simulink model "PrkgClimaActvMgr"  (R2022b) (continued)
== system PrkgClimaActvMgr == (continued)
  MemSaveFlgForBlwAftRun_MemSaveFlgForBlwAftRun : Outport  Port=18  OutDataTypeStr=Boolean  PortDimensions=1
  MemSaveFlgForHighTempCln_MemSaveFlgForHighTempCln : Outport  Port=19  OutDataTypeStr=Boolean  PortDimensions=1
  MemSaveFlgForHMISetEngReq_MemSaveFlgForHMISetEngReq : Outport  Port=20  OutDataTypeStr=Boolean  PortDimensions=1
  MemSaveFlgForParkVent_MemSaveFlgForParkVent : Outport  Port=21  OutDataTypeStr=Boolean  PortDimensions=1
  MemSaveFlgForParkVentActvCnt_MemSaveFlgForParkVentActvCnt : Outport  Port=22  OutDataTypeStr=Boolean  PortDimensions=1
  MemWrForBlwAftRun_MemWrForBlwAftRun : Outport  Port=23  OutDataTypeStr=Bus: MemRecForBlwAftRun  PortDimensions=1  BusOutputAsStruct=on
  MemWrForHighTempCln_MemWrForHighTempCln : Outport  Port=24  OutDataTypeStr=Bus: MemRecForHighTempCln  PortDimensions=1  BusOutputAsStruct=on
  MemWrForHMISetEngReq_MemWrForHMISetEngReq : Outport  Port=25  OutDataTypeStr=Bus: MemRecForHMISetEngReq  PortDimensions=1  BusOutputAsStruct=on
  MemWrForParkVent_MemWrForParkVent : Outport  Port=26  OutDataTypeStr=Bus: MemRecForParkVent  PortDimensions=1  BusOutputAsStruct=on
  MemWrForParkVentActvCnt_MemWrForParkVentActvCnt : Outport  Port=27  OutDataTypeStr=Bus: MemRecForParkVentCnt  PortDimensions=1  BusOutputAsStruct=on
  OGMAirSrcFnshd_OGMAirSrcFnshd : Outport  Port=28  OutDataTypeStr=Boolean  PortDimensions=1
  OGMAirSrcReq_OGMAirSrcReq : Outport  Port=29  OutDataTypeStr=Enum: OnOffNoReq  PortDimensions=1
  OGMAvlSts_OGMAvlSts : Outport  Port=30  OutDataTypeStr=Enum: OxygenSplyAvlSts  PortDimensions=1
  OGMReq_OGMReq : Outport  Port=31  OutDataTypeStr=Enum: OxygenSplyReq  PortDimensions=1
  ParkVentActv_ParkVentActv : Outport  Port=32  OutDataTypeStr=Boolean  PortDimensions=1
  ParkVentWkTi_ParkVentWkTi : Outport  Port=33  OutDataTypeStr=UInt8  PortDimensions=1
  PrkgClimaACReq_PrkgClimaACReq : Outport  Port=34  OutDataTypeStr=Enum: HmiCmptmtCoolgReq  PortDimensions=1
  PrkgClimaActvMgrVFCLDACClimaCtrl_PrkgClimaActvMgrVFCLDACClimaCtrl : Outport  Port=35  OutDataTypeStr=Boolean  PortDimensions=1
  PrkgClimaAirflowReqRe_PrkgClimaAirflowReqRe : Outport  Port=36  OutDataTypeStr=Enum: HmiHvacFanLvl  PortDimensions=1
  PrkgClimaEngStReqNgcc_PrkgClimaEngStReqNgcc : Outport  Port=37  OutDataTypeStr=Enum: OnOff1  PortDimensions=1
  PrkgClimaHVReq_PrkgClimaHVReq : Outport  Port=38  OutDataTypeStr=Enum: OnOff1  PortDimensions=1
  PrkgClimaOnReqRe_PrkgClimaOnReqRe : Outport  Port=39  OutDataTypeStr=Enum: OnOff1  PortDimensions=1
  PrkgCmftRlyReq_PrkgCmftRlyReq : Outport  Port=40  OutDataTypeStr=Boolean  PortDimensions=1
  PrkgOGMAirSrcActv_PrkgOGMAirSrcActv : Outport  Port=41  OutDataTypeStr=Boolean  PortDimensions=1
  PrkgVntFctn_PrkgVntFctn : Outport  Port=42  OutDataTypeStr=Boolean  PortDimensions=1
  PrvntWinFrz_PrvntWinFrz : Outport  Port=43  OutDataTypeStr=Boolean  PortDimensions=1
  PrvntWinFrzFnshd_PrvntWinFrzFnshd : Outport  Port=44  OutDataTypeStr=Boolean  PortDimensions=1
  RemActvSts_RemActvSts : Outport  Port=45  OutDataTypeStr=Bus: RemActvSts  PortDimensions=1  BusOutputAsStruct=on
  RemClimaFnshd1_RemClimaFnshd1 : Outport  Port=46  OutDataTypeStr=Boolean  PortDimensions=1
  RemClimaWarn_RemClimaWarn : Outport  Port=47  OutDataTypeStr=Enum: ClimaWarn  PortDimensions=1
  RemOxygenSplyActv_RemOxygenSplyActv : Outport  Port=48  OutDataTypeStr=Enum: OnOff1  PortDimensions=1
  RemOxygenSplyActvFaildNgcc_RemOxygenSplyActvFaildNgcc : Outport  Port=49  OutMin=0  OutMax=3  OutDataTypeStr=UInt2  PortDimensions=1
  RemOxygenSplyFnshd_RemOxygenSplyFnshd : Outport  Port=50  OutDataTypeStr=Boolean  PortDimensions=1
  RemOxygenSplyFnshd1_RemOxygenSplyFnshd1 : Outport  Port=51  OutDataTypeStr=Boolean  PortDimensions=1
  RemOxygenSplyWarn_RemOxygenSplyWarn : Outport  Port=52  OutDataTypeStr=Enum: ClimaWarn  PortDimensions=1
  RemSynchrnztnLcl_RemSynchrnztnLcl : Outport  Port=53  OutDataTypeStr=Bus: RemSynchrnztnLcl  PortDimensions=1  BusOutputAsStruct=on
  RemWinClsReq_RemWinClsReq : Outport  Port=54  OutDataTypeStr=Enum: NoReqOnOff  PortDimensions=1
  SeatClimaDurgClimaHmiSeatHeatgForRowThrdLe_SeatClimaDurgClimaHmiSeatHeatgForRowThrdLe : Outport  Port=55  OutDataTypeStr=Enum: SeatClimaLvl  PortDimensions=1
  SeatClimaDurgClimaHmiSeatHeatgForRowThrdRi_SeatClimaDurgClimaHmiSeatHeatgForRowThrdRi : Outport  Port=56  OutDataTypeStr=Enum: SeatClimaLvl  PortDimensions=1
  SeatClimaDurgClimaHmiSeatVentnForRowThrdLe_SeatClimaDurgClimaHmiSeatVentnForRowThrdLe : Outport  Port=57  OutDataTypeStr=Enum: SeatClimaLvl  PortDimensions=1
  SeatClimaDurgClimaHmiSeatVentnForRowThrdRi_SeatClimaDurgClimaHmiSeatVentnForRowThrdRi : Outport  Port=58  OutDataTypeStr=Enum: SeatClimaLvl  PortDimensions=1
  TelmDefrostFnshd_TelmDefrostFnshd : Outport  Port=59  OutDataTypeStr=Boolean  PortDimensions=1
  TelmSeatHeatDrvrFnshd_TelmSeatHeatDrvrFnshd : Outport  Port=60  OutDataTypeStr=Boolean  PortDimensions=1
  TelmSeatHeatPassFnshd_TelmSeatHeatPassFnshd : Outport  Port=61  OutDataTypeStr=Boolean  PortDimensions=1
  TelmSeatHeatSecLeFnshd_TelmSeatHeatSecLeFnshd : Outport  Port=62  OutDataTypeStr=Boolean  PortDimensions=1
  TelmSeatHeatSecRiFnshd_TelmSeatHeatSecRiFnshd : Outport  Port=63  OutDataTypeStr=Boolean  PortDimensions=1
  TelmSeatHeatThrdLeFnshd_TelmSeatHeatThrdLeFnshd : Outport  Port=64  OutDataTypeStr=Boolean  PortDimensions=1
  TelmSeatHeatThrdRiFnshd_TelmSeatHeatThrdRiFnshd : Outport  Port=65  OutDataTypeStr=Boolean  PortDimensions=1
  TelmSeatVentnDrvrFnshd_TelmSeatVentnDrvrFnshd : Outport  Port=66  OutDataTypeStr=Boolean  PortDimensions=1
  TelmSeatVentnPassFnshd_TelmSeatVentnPassFnshd : Outport  Port=67  OutDataTypeStr=Boolean  PortDimensions=1
  TelmSeatVentnSecLeFnshd_TelmSeatVentnSecLeFnshd : Outport  Port=68  OutDataTypeStr=Boolean  PortDimensions=1
  TelmSeatVentnSecRiFnshd_TelmSeatVentnSecRiFnshd : Outport  Port=69  OutDataTypeStr=Boolean  PortDimensions=1
  TelmSeatVentnThrdLeFnshd_TelmSeatVentnThrdLeFnshd : Outport  Port=70  OutDataTypeStr=Boolean  PortDimensions=1
  TelmSeatVentnThrdRiFnshd_TelmSeatVentnThrdRiFnshd : Outport  Port=71  OutDataTypeStr=Boolean  PortDimensions=1
  TelmSteerWhlHeatFnshd_TelmSteerWhlHeatFnshd : Outport  Port=72  OutDataTypeStr=Boolean  PortDimensions=1
  VMMChngNdMtnNgcc_VMMChngNdMtnNgcc : Outport  Port=73  OutDataTypeStr=Enum: OnOff1  PortDimensions=1
  VTMOverTProtection_VTMOverTProtection : Outport  Port=74  OutDataTypeStr=Boolean  PortDimensions=1
  VTMOverTProtectionFnshd_VTMOverTProtectionFnshd : Outport  Port=75  OutDataTypeStr=Boolean  PortDimensions=1
  BlwAftRunReq_BlwAftRunReq : Outport  Port=76  OutDataTypeStr=Boolean  PortDimensions=1
  BlwAftRunSts_BlwAftRunSts : Outport  Port=77  OutDataTypeStr=Boolean  PortDimensions=1
  BookTrvlClimaActv_BookTrvlClimaActv : Outport  Port=78  OutDataTypeStr=Enum: OnOff1  PortDimensions=1
  CdsParkgClimaActv_CdsParkgClimaActv : Outport  Port=79  OutDataTypeStr=Enum: OnOff1  PortDimensions=1
  ClimaActv_Ngcc_ClimaActv : Outport  Port=80  OutDataTypeStr=Enum: OnOff1  PortDimensions=1
  ClimaOvrHeatProActv_ClimaOvrHeatProActv : Outport  Port=81  OutDataTypeStr=Enum: OnOff1  PortDimensions=1
  ClimaOvrHeatProActvSts_ClimaOvrHeatProActvSts : Outport  Port=82  OutDataTypeStr=Enum: OnOff1  PortDimensions=1
  ClimaOvrHeatProWarn_ClimaOvrHeatProWarn : Outport  Port=83  OutDataTypeStr=Enum: ClimaOvrheatProWarnSts  PortDimensions=1
  ClimaOvrHeatPrtSts_ClimaOvrHeatPrtSts : Outport  Port=84  OutDataTypeStr=Enum: OnOff1  PortDimensions=1
  ClimaSts_ClimaSts : Outport  Port=85  OutDataTypeStr=Enum: ClimaSts  PortDimensions=1
  ClimaTiOfEnd_Ngcc_ClimaTiOfEnd : Outport  Port=86  OutDataTypeStr=CarTiGlb  PortDimensions=1
  ClimaWarn_ClimaWarn : Outport  Port=87  OutDataTypeStr=Enum: ClimaWarn  PortDimensions=1
  ClngActv_ClngActv : Outport  Port=88  OutDataTypeStr=Enum: OnOffNoReq  PortDimensions=1
  COHSts_COHSts : Outport  Port=89  OutDataTypeStr=Enum: CabinOHSts  PortDimensions=1
  CoolgFastForPrePrkgClimaActv_CoolgFastForPrePrkgClimaActv : Outport  Port=90  OutDataTypeStr=Boolean  PortDimensions=1
  CoolgFastUniqueForPrePrkgClimaActv_CoolgFastUniqueForPrePrkgClimaActv : Outport  Port=91  OutDataTypeStr=Boolean  PortDimensions=1
  DefrstReq_DefrstReq : Outport  Port=92  OutDataTypeStr=Bus: DefrstReq  PortDimensions=1  BusOutputAsStruct=on
  DiDblLock_DiDblLock : Outport  Port=93  OutDataTypeStr=Enum: EnableDisableCoding  PortDimensions=1
  EcuKeepActvFromPrkgClima_EcuKeepActvFromPrkgClima : Outport  Port=94  OutDataTypeStr=Boolean  PortDimensions=1
  ExtVentFanVolStsFrmClima_ExtVentFanVolStsFrmClima : Outport  Port=95  OutDataTypeStr=Enum: Level4  PortDimensions=1
  ExtVentStsFrmClima_ExtVentStsFrmClima : Outport  Port=96  OutDataTypeStr=Enum: OnOff1  PortDimensions=1
  ExtVentToastFrmClima_Prkg_ExtVentToastFrmClima : Outport  Port=97  OutDataTypeStr=Enum: ExtVentToastFrmClimaSts  PortDimensions=1
  HeatrElecFrntReqFromPrkgClima_HeatrElecFrntReqFromPrkgClima : Outport  Port=98  OutDataTypeStr=Boolean  PortDimensions=1
  IntCo2ActvSts_IntCo2ActvSts : Outport  Port=99  OutDataTypeStr=Enum: IntCo2Sts  PortDimensions=1
  KeyClimaActv_KeyClimaActv : Outport  Port=100  OutDataTypeStr=Enum: OnOff1  PortDimensions=1
  MemSaveFlgForPrkgClimaActvMgr_MemSaveFlgForPrkgClimaActvMgr : Outport  Port=101  OutDataTypeStr=Boolean  PortDimensions=1
  ModForPrkgClima_ModForPrkgClima : Outport  Port=102  OutDataTypeStr=Enum: PrkgClimaMod  PortDimensions=1
  PreClngActv_PreClngActv : Outport  Description=|  Port=103  OutDataTypeStr=Enum: OnOffNoReq  PortDimensions=1
      | The CCM shall request PM2.5 measurement during pre cleaning with the signal.
      | 0 = Signal value not valid (default)
      | 1= Pre Cleaning active
      | 2= No request
  PreClngNotif_PreClngNotif : Outport  Port=104  OutDataTypeStr=Enum: PreClngNotif  PortDimensions=1
  PreClngSts_PreClngSts : Outport  Port=105  OutDataTypeStr=Enum: PreClngSts  PortDimensions=1
  PrkgClimaActvMgrVFCBodyPreClimatisation_PrkgClimaActvMgrVFCBodyPreClimatisation : Outport  Port=106  OutDataTypeStr=Boolean  PortDimensions=1
  PrkgClimaActvMgrVFCGlobalShortDuration_PrkgClimaActvMgrVFCGlobalShortDuration : Outport  Port=107  OutDataTypeStr=Boolean  PortDimensions=1
  PrkgClimaActvMgrVFCLocking_PrkgClimaActvMgrVFCLocking : Outport  Port=108  OutDataTypeStr=Boolean  PortDimensions=1
  PrkgClimaActvMgrVFCParkingDrivingClimatization_PrkgClimaActvMgrVFCParkingDrivingClimatization : Outport  Port=109  OutDataTypeStr=Boolean  PortDimensions=1
  PrkgClimaActvMgrVFCPostClimatisation_PrkgClimaActvMgrVFCPostClimatisation : Outport  Port=110  OutDataTypeStr=Boolean  PortDimensions=1
  PrkgClimaActvMgrVFCWindows_PrkgClimaActvMgrVFCWindows : Outport  Port=111  OutDataTypeStr=Boolean  PortDimensions=1
  PrkgClimaAirflowReq_PrkgClimaAirflowReq : Outport  Port=112  OutDataTypeStr=Enum: HmiHvacFanLvl  PortDimensions=1
  PrkgClimaFnshd_PrkgClimaFnshd : Outport  Port=113  OutDataTypeStr=Boolean  PortDimensions=1
  PrkgClimaHeatgReq_PrkgClimaHeatgReq : Outport  Port=114  OutDataTypeStr=Boolean  PortDimensions=1
  PrkgClimaModReq_PrkgClimaModReq : Outport  Port=115  OutDataTypeStr=Enum: HmiCmptmtAirDistbnFrnt  PortDimensions=1
  PrkgClimaOnReq_PrkgClimaOnReq : Outport  Port=116  OutDataTypeStr=Boolean  PortDimensions=1
  PrkgClimaPostCdngReq_PrkgClimaPostCdngReq : Outport  Port=117  OutDataTypeStr=Boolean  PortDimensions=1
  PrkgClimaPreCdngReq_PrkgClimaPreCdngReq : Outport  Port=118  OutDataTypeStr=Boolean  PortDimensions=1
  PrkgClimaRecirReq_PrkgClimaRecirReq : Outport  Port=119  OutDataTypeStr=Enum: HmiHvacRecircCmd  PortDimensions=1
  PrkgClimaRqrdImdtForBattULo_PrkgClimaRqrdImdtForBattULo : Outport  Port=120  OutDataTypeStr=Boolean  PortDimensions=1
  PrkgClimaRqrdImdtFromActvMgr_PrkgClimaRqrdImdtFromActvMgr : Outport  Port=121  OutDataTypeStr=Boolean  PortDimensions=1
  PrkgClimaRqrdTmrForBattULo_PrkgClimaRqrdTmrForBattULo : Outport  Port=122  OutDataTypeStr=Boolean  PortDimensions=1
  PrkgClimaTemp_PrkgClimaTemp : Outport  Port=123  OutDataTypeStr=T30  PortDimensions=1
  PrkgClimaWarn_PrkgClimaWarn : Outport  Port=124  OutDataTypeStr=Enum: ClimaWarn  PortDimensions=1
  PrkgModMgr_PrkgModMgr : Outport  Port=125  OutDataTypeStr=Enum: PrkgModMgr  PortDimensions=1
  RemClimaActv_RemClimaActv : Outport  Port=126  OutDataTypeStr=Enum: OnOff1  PortDimensions=1
  RemClimaDefrstSts_RemClimaDefrstSts : Outport  Port=127  OutDataTypeStr=Enum: OnOff1  PortDimensions=1
  RemClimaDelaySts_RemClimaDelaySts : Outport  Port=128  OutDataTypeStr=Enum: OnOff1  PortDimensions=1
  RemClimaEgyFinalReq_RemClimaEgyFinalReq : Outport  Port=129  OutDataTypeStr=UInt32  PortDimensions=1
  RemClimaExtnTiRspn_RemClimaExtnTiRspn : Outport  Port=130  OutDataTypeStr=Enum: OnOff1  PortDimensions=1
  RemClimaFnshd_RemClimaFnshd : Outport  Port=131  OutDataTypeStr=Boolean  PortDimensions=1
  RemClimaHvSts_RemClimaHvSts : Outport  Port=132  OutDataTypeStr=Enum: OnOff1  PortDimensions=1
  RemStrtClimaRspn_RemStrtClimaRspn : Outport  Port=133  OutDataTypeStr=Enum: OnOff1  PortDimensions=1
  RemVentActvSts_RemVentActvSts : Outport  Port=134  OutDataTypeStr=Enum: OnOff1  PortDimensions=1
  RemVentFbSts_RemVentFbSts : Outport  Port=135  OutDataTypeStr=Enum: RemVentSts  PortDimensions=1
  RemVentReqRspnFb_RemVentReqRspnFb : Outport  Port=136  OutDataTypeStr=Enum: OnOff1  PortDimensions=1
  RemVentWarnSts_RemVentWarnSts : Outport  Port=137  OutDataTypeStr=Enum: RemVentWarningSts  PortDimensions=1
  ReqForPrkgClima_ReqForPrkgClima : Outport  Port=138  OutDataTypeStr=Enum: PrkgClimaReqSts  PortDimensions=1
  ReqForSwtHeatrTypFromActvMgr_ReqForSwtHeatrTypFromActvMgr : Outport  Port=139  OutDataTypeStr=Boolean  PortDimensions=1
  RoofOpnReqFrmClima_RoofOpnReqFrmClima : Outport  Port=140  OutDataTypeStr=Enum: OnOff1  PortDimensions=1
  RtLeForPrkgClima_RtLeForPrkgClima : Outport  Port=141  OutDataTypeStr=Ti33  PortDimensions=1
  SchedCmptHeatCoolFB_SchedCmptHeatCoolFB : Outport  Port=142  OutDataTypeStr=Enum: SchedCmptHeatCoolFB  PortDimensions=1
  SeatClimaDurgClima_SeatClimaDurgClima : Outport  Port=143  OutDataTypeStr=Bus: HmiSeatClima  PortDimensions=1  BusOutputAsStruct=on
  SteerWhlHeatgDurgClima_Ngcc_SteerWhlHeatgDurgClima : Outport  Port=144  OutDataTypeStr=Boolean  PortDimensions=1
  SuspStrtActvForPrkgClima_SuspStrtActvForPrkgClima : Outport  Port=145  OutDataTypeStr=Boolean  PortDimensions=1
  WarnForHybWoUFromActvMgr_WarnForHybWoUFromActvMgr : Outport  Port=146  OutDataTypeStr=Boolean  PortDimensions=1
  wires:
  ActvRqrdForPostPrkgClima_ActvRqrdForPostPrkgClima/1 -> PrkgClimaActvMgr_Runnable_sys/1(ActvRqrdForPostPrkgClima_ActvRqrdForPostPrkgClima_read)
  CarTiIntForClima_CarTiIntForClima/1 -> PrkgClimaActvMgr_Runnable_sys/2(CarTiIntForClima_CarTiIntForClima_read)
  CdsClimaOnReqfromExsrc_CdsClimaOnReqfromExsrc/1 -> PrkgClimaActvMgr_Runnable_sys/3(CdsClimaOnReqfromExsrc_CdsClimaOnReqfromExsrc_read)
  ClimaCfgForClimaCtrlMan1_ClimaCfgForClimaCtrlMan1/1 -> PrkgClimaActvMgr_Runnable_sys/4(ClimaCfgForClimaCtrlMan1_ClimaCfgForClimaCtrlMan1_read)
  ClimaCfgForCmptmtClng_ClimaCfgForCmptmtClng/1 -> PrkgClimaActvMgr_Runnable_sys/5(ClimaCfgForCmptmtClng_ClimaCfgForCmptmtClng_read)
  ClimaCfgForFoldCSD_ClimaCfgForFoldCSD/1 -> PrkgClimaActvMgr_Runnable_sys/6(ClimaCfgForFoldCSD_ClimaCfgForFoldCSD_read)
  ClimaCfgForNrOfTSetg1_ClimaCfgForNrOfTSetg1/1 -> PrkgClimaActvMgr_Runnable_sys/7(ClimaCfgForNrOfTSetg1_ClimaCfgForNrOfTSetg1_read)
  ClimaCfgForPreClng1_ClimaCfgForPreClng1/1 -> PrkgClimaActvMgr_Runnable_sys/8(ClimaCfgForPreClng1_ClimaCfgForPreClng1_read)
  ClimaCfgForSeatHeatgForRowFirstPrsnt1_ClimaCfgForSeatHeatgForRowFirstPrsnt1/1 -> PrkgClimaActvMgr_Runnable_sys/9(ClimaCfgForSeatHeatgForRowFirstPrsnt1_ClimaCfgForSeatHeatgForRowFirstPrsnt1_read)
  ClimaCfgForSeatHeatgForRowSec_ClimaCfgForSeatHeatgForRowSec/1 -> PrkgClimaActvMgr_Runnable_sys/10(ClimaCfgForSeatHeatgForRowSec_ClimaCfgForSeatHeatgForRowSec_read)
  ClimaCfgForSeatVentnForRowFirst_ClimaCfgForSeatVentnForRowFirst/1 -> PrkgClimaActvMgr_Runnable_sys/11(ClimaCfgForSeatVentnForRowFirst_ClimaCfgForSeatVentnForRowFirst_read)
  ClimaCfgForSeatVentnForRowSec_ClimaCfgForSeatVentnForRowSec/1 -> PrkgClimaActvMgr_Runnable_sys/12(ClimaCfgForSeatVentnForRowSec_ClimaCfgForSeatVentnForRowSec_read)
  ClimaCfgForSteerWhlHeatgPrsnt1_ClimaCfgForSteerWhlHeatgPrsnt1/1 -> PrkgClimaActvMgr_Runnable_sys/13(ClimaCfgForSteerWhlHeatgPrsnt1_ClimaCfgForSteerWhlHeatgPrsnt1_read)
  ClimaCfgForSteerWhlLeSide1_ClimaCfgForSteerWhlLeSide1/1 -> PrkgClimaActvMgr_Runnable_sys/14(ClimaCfgForSteerWhlLeSide1_ClimaCfgForSteerWhlLeSide1_read)
  ClimaCfgForVehElec1_ClimaCfgForVehElec1/1 -> PrkgClimaActvMgr_Runnable_sys/15(ClimaCfgForVehElec1_ClimaCfgForVehElec1_read)
  ClimaCfgForVehHyb1_ClimaCfgForVehHyb1/1 -> PrkgClimaActvMgr_Runnable_sys/16(ClimaCfgForVehHyb1_ClimaCfgForVehHyb1_read)
  ClimaCfgForWindHeatrPrsnt1_ClimaCfgForWindHeatrPrsnt1/1 -> PrkgClimaActvMgr_Runnable_sys/17(ClimaCfgForWindHeatrPrsnt1_ClimaCfgForWindHeatrPrsnt1_read)
  ClimaOvrHeatProWarnIni_ClimaOvrHeatProWarnIni/1 -> PrkgClimaActvMgr_Runnable_sys/18(ClimaOvrHeatProWarnIni_ClimaOvrHeatProWarnIni_read)
  ClimaOvrHeatReqFromExrsc_ClimaOvrHeatReqFromExrsc/1 -> PrkgClimaActvMgr_Runnable_sys/19(ClimaOvrHeatReqFromExrsc_ClimaOvrHeatReqFromExrsc_read)
  ClimaOvrHeatStrtReq_ClimaOvrHeatStrtReq/1 -> PrkgClimaActvMgr_Runnable_sys/20(ClimaOvrHeatStrtReq_ClimaOvrHeatStrtReq_read)
  CmptmtClsd_CmptmtClsd/1 -> PrkgClimaActvMgr_Runnable_sys/21(CmptmtClsd_CmptmtClsd_read)
  CSDUsgChgeOffReq_CSDUsgChgeOffReq/1 -> PrkgClimaActvMgr_Runnable_sys/22(CSDUsgChgeOffReq_CSDUsgChgeOffReq_read)
  CSDUsgChgeOnReq_CSDUsgChgeOnReq/1 -> PrkgClimaActvMgr_Runnable_sys/23(CSDUsgChgeOnReq_CSDUsgChgeOnReq_read)
  DefrstHeatgForPrkgClimaEnad_DefrstHeatgForPrkgClimaEnad/1 -> PrkgClimaActvMgr_Runnable_sys/24(DefrstHeatgForPrkgClimaEnad_DefrstHeatgForPrkgClimaEnad_read)
  DoorOpenStsForClima_DoorOpenStsForClima/1 -> PrkgClimaActvMgr_Runnable_sys/25(DoorOpenStsForClima_DoorOpenStsForClima_read)
  EgyAllwdForPrkgClima_EgyAllwdForPrkgClima/1 -> PrkgClimaActvMgr_Runnable_sys/26(EgyAllwdForPrkgClima_EgyAllwdForPrkgClima_read)
  EgyPrioForPrkgClima_EgyPrioForPrkgClima/1 -> PrkgClimaActvMgr_Runnable_sys/27(EgyPrioForPrkgClima_EgyPrioForPrkgClima_read)
  ErsCmdByKeyStrtReq_ErsCmdByKeyStrtReq/1 -> PrkgClimaActvMgr_Runnable_sys/28(ErsCmdByKeyStrtReq_ErsCmdByKeyStrtReq_read)
  ErsRunTimelStrtReq_ErsRunTimelStrtReq/1 -> PrkgClimaActvMgr_Runnable_sys/29(ErsRunTimelStrtReq_ErsRunTimelStrtReq_read)
  FacForRtForPrkgClima_FacForRtForPrkgClima/1 -> PrkgClimaActvMgr_Runnable_sys/30(FacForRtForPrkgClima_FacForRtForPrkgClima_read)
  FrntHvacBlowerSts_FrntHvacBlowerSts/1 -> PrkgClimaActvMgr_Runnable_sys/31(FrntHvacBlowerSts_FrntHvacBlowerSts_read)
  HeatrTypForPrkgClima_HeatrTypForPrkgClima/1 -> PrkgClimaActvMgr_Runnable_sys/32(HeatrTypForPrkgClima_HeatrTypForPrkgClima_read)
  HeatUpRqrdForPrkgClima_HeatUpRqrdForPrkgClima/1 -> PrkgClimaActvMgr_Runnable_sys/33(HeatUpRqrdForPrkgClima_HeatUpRqrdForPrkgClima_read)
  HMIExtVentFanVolReqStrtReq_HMIExtVentFanVolReqStrtReq/1 -> PrkgClimaActvMgr_Runnable_sys/34(HMIExtVentFanVolReqStrtReq_HMIExtVentFanVolReqStrtReq_read)
  HMIExtVentonoffReqStrtReq_HMIExtVentonoffReqStrtReq/1 -> PrkgClimaActvMgr_Runnable_sys/35(HMIExtVentonoffReqStrtReq_HMIExtVentonoffReqStrtReq_read)
  HMIExtVentTSpReqStrtReq_HMIExtVentTSpReqStrtReq/1 -> PrkgClimaActvMgr_Runnable_sys/36(HMIExtVentTSpReqStrtReq_HMIExtVentTSpReqStrtReq_read)
  HMIExtVentTStsReqStrtReq_HMIExtVentTStsReqStrtReq/1 -> PrkgClimaActvMgr_Runnable_sys/37(HMIExtVentTStsReqStrtReq_HMIExtVentTStsReqStrtReq_read)
  HvBattForPrkgClima_HvBattForPrkgClima/1 -> PrkgClimaActvMgr_Runnable_sys/38(HvBattForPrkgClima_HvBattForPrkgClima_read)
  HvBattSoc_HvBattSoc/1 -> PrkgClimaActvMgr_Runnable_sys/39(HvBattSoc_HvBattSoc_read)
  HvSysCnctWoU_HvSysCnctWoU/1 -> PrkgClimaActvMgr_Runnable_sys/40(HvSysCnctWoU_HvSysCnctWoU_read)
  HvSysWoU_HvSysWoU/1 -> PrkgClimaActvMgr_Runnable_sys/41(HvSysWoU_HvSysWoU_read)
  HvSysRlySts_HvSysRlySts/1 -> PrkgClimaActvMgr_Runnable_sys/42(HvSysRlySts_HvSysRlySts_read)
  IntCo2MontrStrtReq_IntCo2MontrStrtReq/1 -> PrkgClimaActvMgr_Runnable_sys/43(IntCo2MontrStrtReq_IntCo2MontrStrtReq_read)
  LockgCenSts_Ngcc_LockgCenSts/1 -> PrkgClimaActvMgr_Runnable_sys/44(LockgCenSts_Ngcc_LockgCenSts_read)
  MemReadForPrkgClimaActvMgr_MemReadForPrkgClimaActvMgr/1 -> PrkgClimaActvMgr_Runnable_sys/45(MemReadForPrkgClimaActvMgr_MemReadForPrkgClimaActvMgr_read)
  MemReqStsForPrkgClima_MemReqStsForPrkgClima/1 -> PrkgClimaActvMgr_Runnable_sys/46(MemReqStsForPrkgClima_MemReqStsForPrkgClima_read)
  OffForTLoForPostCdng_OffForTLoForPostCdng/1 -> PrkgClimaActvMgr_Runnable_sys/47(OffForTLoForPostCdng_OffForTLoForPostCdng_read)
  OffRqrdForPrkgClima_OffRqrdForPrkgClima/1 -> PrkgClimaActvMgr_Runnable_sys/48(OffRqrdForPrkgClima_OffRqrdForPrkgClima_read)
  PrePrkgClimaNotAvlForHvSysWoU_PrePrkgClimaNotAvlForHvSysWoU/1 -> PrkgClimaActvMgr_Runnable_sys/49(PrePrkgClimaNotAvlForHvSysWoU_PrePrkgClimaNotAvlForHvSysWoU_read)
  PrkgCDSAvl_PrkgCDSAvl/1 -> PrkgClimaActvMgr_Runnable_sys/50(PrkgCDSAvl_PrkgCDSAvl_read)
  PrkgClimaAllwdForBattULo_PrkgClimaAllwdForBattULo/1 -> PrkgClimaActvMgr_Runnable_sys/51(PrkgClimaAllwdForBattULo_PrkgClimaAllwdForBattULo_read)
  PrkgClimaAllwdForSwtHeatrTyp_PrkgClimaAllwdForSwtHeatrTyp/1 -> PrkgClimaActvMgr_Runnable_sys/52(PrkgClimaAllwdForSwtHeatrTyp_PrkgClimaAllwdForSwtHeatrTyp_read)
  PrkgHeatgActv_PrkgHeatgActv/1 -> PrkgClimaActvMgr_Runnable_sys/53(PrkgHeatgActv_PrkgHeatgActv_read)
  PwrAllwdForHvacFanFrnt_PwrAllwdForHvacFanFrnt/1 -> PrkgClimaActvMgr_Runnable_sys/54(PwrAllwdForHvacFanFrnt_PwrAllwdForHvacFanFrnt_read)
  PwrCnsDesForHvacFanFrnt_PwrCnsDesForHvacFanFrnt/1 -> PrkgClimaActvMgr_Runnable_sys/55(PwrCnsDesForHvacFanFrnt_PwrCnsDesForHvacFanFrnt_read)
  RemClimaallExsrcReq_RemClimaallExsrcReq/1 -> PrkgClimaActvMgr_Runnable_sys/56(RemClimaallExsrcReq_RemClimaallExsrcReq_read)
  RemClimaEgyExsrcReq_RemClimaEgyExsrcReq/1 -> PrkgClimaActvMgr_Runnable_sys/57(RemClimaEgyExsrcReq_RemClimaEgyExsrcReq_read)
  RemClimaTmrStrtReq_RemClimaTmrStrtReq/1 -> PrkgClimaActvMgr_Runnable_sys/58(RemClimaTmrStrtReq_RemClimaTmrStrtReq_read)
  RemStrtExtnTiStrtReq_RemStrtExtnTiStrtReq/1 -> PrkgClimaActvMgr_Runnable_sys/59(RemStrtExtnTiStrtReq_RemStrtExtnTiStrtReq_read)
  RemStrtHvCtrlStrtReq_RemStrtHvCtrlStrtReq/1 -> PrkgClimaActvMgr_Runnable_sys/60(RemStrtHvCtrlStrtReq_RemStrtHvCtrlStrtReq_read)
  RemVentReqStrtReq_RemVentReqStrtReq/1 -> PrkgClimaActvMgr_Runnable_sys/61(RemVentReqStrtReq_RemVentReqStrtReq_read)
  RtCmftForImdtPrePrkgClima_RtCmftForImdtPrePrkgClima/1 -> PrkgClimaActvMgr_Runnable_sys/62(RtCmftForImdtPrePrkgClima_RtCmftForImdtPrePrkgClima_read)
  RtCmftForPostPrkgClima_RtCmftForPostPrkgClima/1 -> PrkgClimaActvMgr_Runnable_sys/63(RtCmftForPostPrkgClima_RtCmftForPostPrkgClima_read)
  RtCmftForPreClng_RtCmftForPreClng/1 -> PrkgClimaActvMgr_Runnable_sys/64(RtCmftForPreClng_RtCmftForPreClng_read)
  RtSdlForPrkgClima_RtSdlForPrkgClima/1 -> PrkgClimaActvMgr_Runnable_sys/65(RtSdlForPrkgClima_RtSdlForPrkgClima_read)
  SeatHeatForPrkgClimaEnad_SeatHeatForPrkgClimaEnad/1 -> PrkgClimaActvMgr_Runnable_sys/66(SeatHeatForPrkgClimaEnad_SeatHeatForPrkgClimaEnad_read)
  SteerWhlHeatgForPrkgClimaEnad_SteerWhlHeatgForPrkgClimaEnad/1 -> PrkgClimaActvMgr_Runnable_sys/67(SteerWhlHeatgForPrkgClimaEnad_SteerWhlHeatgForPrkgClimaEnad_read)
  StrtRemSts1WdStrtRemSts_Ngcc_StrtRemSts1WdStrtRemSts/1 -> PrkgClimaActvMgr_Runnable_sys/68(StrtRemSts1WdStrtRemSts_Ngcc_StrtRemSts1WdStrtRemSts_read)
  StrtRqrdForDirForPrePrkgClima_StrtRqrdForDirForPrePrkgClima/1 -> PrkgClimaActvMgr_Runnable_sys/69(StrtRqrdForDirForPrePrkgClima_StrtRqrdForDirForPrePrkgClima_read)
  StrtRqrdForPreClng_StrtRqrdForPreClng/1 -> PrkgClimaActvMgr_Runnable_sys/70(StrtRqrdForPreClng_StrtRqrdForPreClng_read)
  StrtRqrdForTmrForPrePrkgClima_StrtRqrdForTmrForPrePrkgClima/1 -> PrkgClimaActvMgr_Runnable_sys/71(StrtRqrdForTmrForPrePrkgClima_StrtRqrdForTmrForPrePrkgClima_read)
  SuspStrtAllwdForPrkgClima_SuspStrtAllwdForPrkgClima/1 -> PrkgClimaActvMgr_Runnable_sys/72(SuspStrtAllwdForPrkgClima_SuspStrtAllwdForPrkgClima_read)
  TelmBookClimaReqHmiCmptmtTSpSpclStrtReq_TelmBookClimaReqHmiCmptmtTSpSpclStrtReq/1 -> PrkgClimaActvMgr_Runnable_sys/73(TelmBookClimaReqHmiCmptmtTSpSpclStrtReq_TelmBookClimaReqHmiCmptmtTSpSpclStrtReq_read)
  TelmBookClimaReqOnOffNoReqStrtReq_TelmBookClimaReqOnOffNoReqStrtReq/1 -> PrkgClimaActvMgr_Runnable_sys/74(TelmBookClimaReqOnOffNoReqStrtReq_TelmBookClimaReqOnOffNoReqStrtReq_read)
  TelmBookClimaReqTempRangeStrtReq_TelmBookClimaReqTempRangeStrtReq/1 -> PrkgClimaActvMgr_Runnable_sys/75(TelmBookClimaReqTempRangeStrtReq_TelmBookClimaReqTempRangeStrtReq_read)
  TelmBookClimaTmrStrtReq_TelmBookClimaTmrStrtReq/1 -> PrkgClimaActvMgr_Runnable_sys/76(TelmBookClimaTmrStrtReq_TelmBookClimaTmrStrtReq_read)
  TelmBookSeatHeatClimalReqDrvHeatClimaLvlStrtReq_TelmBookSeatHeatClimalReqDrvHeatClimaLvlStrtReq/1 -> PrkgClimaActvMgr_Runnable_sys/77(TelmBookSeatHeatClimalReqDrvHeatClimaLvlStrtReq_TelmBookSeatHeatClimalReqDrvHeatClimaLvlStrtReq_read)
  TelmBookSeatHeatClimalReqPassHeatClimaLvlStrtReq_TelmBookSeatHeatClimalReqPassHeatClimaLvlStrtReq/1 -> PrkgClimaActvMgr_Runnable_sys/78(TelmBookSeatHeatClimalReqPassHeatClimaLvlStrtReq_TelmBookSeatHeatClimalReqPassHeatClimaLvlStrtReq_read)
  TelmBookSeatHeatClimalReqSecLeHeatClimaLvlStrtReq_TelmBookSeatHeatClimalReqSecLeHeatClimaLvlStrtReq/1 -> PrkgClimaActvMgr_Runnable_sys/79(TelmBookSeatHeatClimalReqSecLeHeatClimaLvlStrtReq_TelmBookSeatHeatClimalReqSecLeHeatClimaLvlStrtReq_read)
  TelmBookSeatHeatClimalReqSecRiHeatClimaLvlStrtReq_TelmBookSeatHeatClimalReqSecRiHeatClimaLvlStrtReq/1 -> PrkgClimaActvMgr_Runnable_sys/80(TelmBookSeatHeatClimalReqSecRiHeatClimaLvlStrtReq_TelmBookSeatHeatClimalReqSecRiHeatClimaLvlStrtReq_read)
  TelmBookSeatHeatClimalReqThrdLeHeatClimaLvlStrtReq_TelmBookSeatHeatClimalReqThrdLeHeatClimaLvlStrtReq/1 -> PrkgClimaActvMgr_Runnable_sys/81(TelmBookSeatHeatClimalReqThrdLeHeatClimaLvlStrtReq_TelmBookSeatHeatClimalReqThrdLeHeatClimaLvlStrtReq_read)
  TelmBookSeatHeatClimalReqThrdRiHeatClimaLvlStrtReq_TelmBookSeatHeatClimalReqThrdRiHeatClimaLvlStrtReq/1 -> PrkgClimaActvMgr_Runnable_sys/82(TelmBookSeatHeatClimalReqThrdRiHeatClimaLvlStrtReq_TelmBookSeatHeatClimalReqThrdRiHeatClimaLvlStrtReq_read)
  TelmBookSeatVentnClimalReqDrvVentnClimaLvlStrtReq_TelmBookSeatVentnClimalReqDrvVentnClimaLvlStrtReq/1 -> PrkgClimaActvMgr_Runnable_sys/83(TelmBookSeatVentnClimalReqDrvVentnClimaLvlStrtReq_TelmBookSeatVentnClimalReqDrvVentnClimaLvlStrtReq_read)
  TelmBookSeatVentnClimalReqPassVentnClimaLvlStrtReq_TelmBookSeatVentnClimalReqPassVentnClimaLvlStrtReq/1 -> PrkgClimaActvMgr_Runnable_sys/84(TelmBookSeatVentnClimalReqPassVentnClimaLvlStrtReq_TelmBookSeatVentnClimalReqPassVentnClimaLvlStrtReq_read)
  TelmBookSeatVentnClimalReqSecLeVentnClimaLvlStrtReq_TelmBookSeatVentnClimalReqSecLeVentnClimaLvlStrtReq/1 -> PrkgClimaActvMgr_Runnable_sys/85(TelmBookSeatVentnClimalReqSecLeVentnClimaLvlStrtReq_TelmBookSeatVentnClimalReqSecLeVentnClimaLvlStrtReq_read)
  TelmBookSeatVentnClimalReqSecRiVentnClimaLvlStrtReq_TelmBookSeatVentnClimalReqSecRiVentnClimaLvlStrtReq/1 -> PrkgClimaActvMgr_Runnable_sys/86(TelmBookSeatVentnClimalReqSecRiVentnClimaLvlStrtReq_TelmBookSeatVentnClimalReqSecRiVentnClimaLvlStrtReq_read)
  TelmBookSeatVentnClimalReqThrdLeVentnClimaLvlStrtReq_TelmBookSeatVentnClimalReqThrdLeVentnClimaLvlStrtReq/1 -> PrkgClimaActvMgr_Runnable_sys/87(TelmBookSeatVentnClimalReqThrdLeVentnClimaLvlStrtReq_TelmBookSeatVentnClimalReqThrdLeVentnClimaLvlStrtReq_read)
  TelmBookSeatVentnClimalReqThrdRiVentnClimaLvlStrtReq_TelmBookSeatVentnClimalReqThrdRiVentnClimaLvlStrtReq/1 -> PrkgClimaActvMgr_Runnable_sys/88(TelmBookSeatVentnClimalReqThrdRiVentnClimaLvlStrtReq_TelmBookSeatVentnClimalReqThrdRiVentnClimaLvlStrtReq_read)
  TelmBookSteerWhlHeatClimalReqStrtReq_TelmBookSteerWhlHeatClimalReqStrtReq/1 -> PrkgClimaActvMgr_Runnable_sys/89(TelmBookSteerWhlHeatClimalReqStrtReq_TelmBookSteerWhlHeatClimalReqStrtReq_read)
  TelmClimaExtVentnLvlStrtReq_TelmClimaExtVentnLvlStrtReq/1 -> PrkgClimaActvMgr_Runnable_sys/90(TelmClimaExtVentnLvlStrtReq_TelmClimaExtVentnLvlStrtReq_read)
  TelmClimaExtVentnReqStrtReq_TelmClimaExtVentnReqStrtReq/1 -> PrkgClimaActvMgr_Runnable_sys/91(TelmClimaExtVentnReqStrtReq_TelmClimaExtVentnReqStrtReq_read)
  TelmClimaExtVentnTempRangeStrtReq_TelmClimaExtVentnTempRangeStrtReq/1 -> PrkgClimaActvMgr_Runnable_sys/92(TelmClimaExtVentnTempRangeStrtReq_TelmClimaExtVentnTempRangeStrtReq_read)
  TelmClimaExtVentnTSpSpclStrtReq_TelmClimaExtVentnTSpSpclStrtReq/1 -> PrkgClimaActvMgr_Runnable_sys/93(TelmClimaExtVentnTSpSpclStrtReq_TelmClimaExtVentnTSpSpclStrtReq_read)
  UpdRtTotForTmrForPrePrkgClima_UpdRtTotForTmrForPrePrkgClima/1 -> PrkgClimaActvMgr_Runnable_sys/94(UpdRtTotForTmrForPrePrkgClima_UpdRtTotForTmrForPrePrkgClima_read)
  VehModMngtGlbSafe1_Ngcc_VehModMngtGlbSafe1/1 -> PrkgClimaActvMgr_Runnable_sys/95(VehModMngtGlbSafe1_Ngcc_VehModMngtGlbSafe1_read)
  VFCBodyPreClimatisationHmiHumCtrl_VFCBodyPreClimatisationHmiHumCtrl/1 -> PrkgClimaActvMgr_Runnable_sys/96(VFCBodyPreClimatisationHmiHumCtrl_VFCBodyPreClimatisationHmiHumCtrl_read)
  VFCHmiClimaCtrlAct_VFCHmiClimaCtrlAct/1 -> PrkgClimaActvMgr_Runnable_sys/97(VFCHmiClimaCtrlAct_VFCHmiClimaCtrlAct_read)
  VFCPrkDrvgClimabyFragAct_VFCPrkDrvgClimabyFragAct/1 -> PrkgClimaActvMgr_Runnable_sys/98(VFCPrkDrvgClimabyFragAct_VFCPrkDrvgClimabyFragAct_read)
  WarnForEgyAllwdLimd_WarnForEgyAllwdLimd/1 -> PrkgClimaActvMgr_Runnable_sys/99(WarnForEgyAllwdLimd_WarnForEgyAllwdLimd_read)
  WarnForHybWoU_WarnForHybWoU/1 -> PrkgClimaActvMgr_Runnable_sys/100(WarnForHybWoU_WarnForHybWoU_read)
  WarnForPreCdngFctNotAvl_WarnForPreCdngFctNotAvl/1 -> PrkgClimaActvMgr_Runnable_sys/101(WarnForPreCdngFctNotAvl_WarnForPreCdngFctNotAvl_read)
  WarnForPrkgClimaNotAllwd_WarnForPrkgClimaNotAllwd/1 -> PrkgClimaActvMgr_Runnable_sys/102(WarnForPrkgClimaNotAllwd_WarnForPrkgClimaNotAllwd_read)
  WindIceDetdFrnt_Ngcc_WindIceDetdFrnt/1 -> PrkgClimaActvMgr_Runnable_sys/103(WindIceDetdFrnt_Ngcc_WindIceDetdFrnt_read)
  WindIceDetdRe_Ngcc_WindIceDetdRe/1 -> PrkgClimaActvMgr_Runnable_sys/104(WindIceDetdRe_Ngcc_WindIceDetdRe_read)
  AirCdnAvl_AirCdnAvl/1 -> PrkgClimaActvMgr_Runnable_sys/105(AirCdnAvl_AirCdnAvl_read)
  AmbTFildForClimaNgcc_AmbTFildForClima/1 -> PrkgClimaActvMgr_Runnable_sys/106(AmbTFildForClimaNgcc_AmbTFildForClima_read)
  OffRqrdForRemClima_OffRqrdForRemClima/1 -> PrkgClimaActvMgr_Runnable_sys/107(OffRqrdForRemClima_OffRqrdForRemClima_read)
  RemClimaTempStrtReq_RemClimaTempStrtReq/1 -> PrkgClimaActvMgr_Runnable_sys/108(RemClimaTempStrtReq_RemClimaTempStrtReq_read)
  VFCAutDemstOperSts_VFCAutDemstOperSts/1 -> PrkgClimaActvMgr_Runnable_sys/109(VFCAutDemstOperSts_VFCAutDemstOperSts_read)
  VFCPrkDrvgClimabyPM25_VFCPrkDrvgClimabyPM25/1 -> PrkgClimaActvMgr_Runnable_sys/110(VFCPrkDrvgClimabyPM25_VFCPrkDrvgClimabyPM25_read)
  AirCdnAvlRe_AirCdnAvlRe/1 -> PrkgClimaActvMgr_Runnable_sys/111(AirCdnAvlRe_AirCdnAvlRe_read)
  AirSrcStsFbNgcc_AirSrcStsFbNgcc/1 -> PrkgClimaActvMgr_Runnable_sys/112(AirSrcStsFbNgcc_AirSrcStsFbNgcc_read)
  BookTrvlClimaActvReqfromExsrc_BookTrvlClimaActvReqfromExsrc/1 -> PrkgClimaActvMgr_Runnable_sys/113(BookTrvlClimaActvReqfromExsrc_BookTrvlClimaActvReqfromExsrc_read)
  CCMWakeupFunction_CCMWakeupFunction/1 -> PrkgClimaActvMgr_Runnable_sys/114(CCMWakeupFunction_CCMWakeupFunction_read)
  ClimaCfgFor3RDROWSEATHEAT_ClimaCfgFor3RDROWSEATHEAT/1 -> PrkgClimaActvMgr_Runnable_sys/115(ClimaCfgFor3RDROWSEATHEAT_ClimaCfgFor3RDROWSEATHEAT_read)
  ClimaCfgFor3RDROWSEATVENTILATED_ClimaCfgFor3RDROWSEATVENTILATED/1 -> PrkgClimaActvMgr_Runnable_sys/116(ClimaCfgFor3RDROWSEATVENTILATED_ClimaCfgFor3RDROWSEATVENTILATED_read)
  ClimaCfgForAirMtrlSnsrPrsnt1_ClimaCfgForAirMtrlSnsrPrsnt1/1 -> PrkgClimaActvMgr_Runnable_sys/117(ClimaCfgForAirMtrlSnsrPrsnt1_ClimaCfgForAirMtrlSnsrPrsnt1_read)
  ClimaCfgForBRAND_ClimaCfgForBRAND/1 -> PrkgClimaActvMgr_Runnable_sys/118(ClimaCfgForBRAND_ClimaCfgForBRAND_read)
  ClimaCfgForDrvrSeatVentWthSmf2_ClimaCfgForDrvrSeatVentWthSmf2/1 -> PrkgClimaActvMgr_Runnable_sys/119(ClimaCfgForDrvrSeatVentWthSmf2_ClimaCfgForDrvrSeatVentWthSmf2_read)
  ClimaCfgForErev1_ClimaCfgForErev1/1 -> PrkgClimaActvMgr_Runnable_sys/120(ClimaCfgForErev1_ClimaCfgForErev1_read)
  ClimaCfgForHvacRePrsnt1_ClimaCfgForHvacRePrsnt1/1 -> PrkgClimaActvMgr_Runnable_sys/121(ClimaCfgForHvacRePrsnt1_ClimaCfgForHvacRePrsnt1_read)
  ClimaCfgForHvacTyp_ClimaCfgForHvacTyp/1 -> PrkgClimaActvMgr_Runnable_sys/122(ClimaCfgForHvacTyp_ClimaCfgForHvacTyp_read)
  ClimaCfgForLifeDetection_ClimaCfgForLifeDetection/1 -> PrkgClimaActvMgr_Runnable_sys/123(ClimaCfgForLifeDetection_ClimaCfgForLifeDetection_read)
  ClimaCfgForMARKET_ClimaCfgForMARKET/1 -> PrkgClimaActvMgr_Runnable_sys/124(ClimaCfgForMARKET_ClimaCfgForMARKET_read)
  ClimaCfgForPassSeatVentWthSmf2_ClimaCfgForPassSeatVentWthSmf2/1 -> PrkgClimaActvMgr_Runnable_sys/125(ClimaCfgForPassSeatVentWthSmf2_ClimaCfgForPassSeatVentWthSmf2_read)
  ClimaCfgForPrpsnTyp_ClimaCfgForPrpsnTyp/1 -> PrkgClimaActvMgr_Runnable_sys/126(ClimaCfgForPrpsnTyp_ClimaCfgForPrpsnTyp_read)
  ClimaCfgForRoofHatch_ClimaCfgForRoofHatch/1 -> PrkgClimaActvMgr_Runnable_sys/127(ClimaCfgForRoofHatch_ClimaCfgForRoofHatch_read)
  ClimaCfgForVehTyp_ClimaCfgForVehTyp/1 -> PrkgClimaActvMgr_Runnable_sys/128(ClimaCfgForVehTyp_ClimaCfgForVehTyp_read)
  ClimaCfgReqVersionReplace_ClimaCfgReqVersionReplace/1 -> PrkgClimaActvMgr_Runnable_sys/129(ClimaCfgReqVersionReplace_ClimaCfgReqVersionReplace_read)
  CmptmtClngActv_CmptmtClngActv/1 -> PrkgClimaActvMgr_Runnable_sys/130(CmptmtClngActv_CmptmtClngActv_read)
  CmptmtOpenStsForClima_CmptmtOpenStsForClima/1 -> PrkgClimaActvMgr_Runnable_sys/131(CmptmtOpenStsForClima_CmptmtOpenStsForClima_read)
  DoorStsWithOutTrForClima_DoorStsWithOutTrForClima/1 -> PrkgClimaActvMgr_Runnable_sys/132(DoorStsWithOutTrForClima_DoorStsWithOutTrForClima_read)
  DrvrSeatHeatgLvlSts_DrvrSeatHeatgLvlSts/1 -> PrkgClimaActvMgr_Runnable_sys/133(DrvrSeatHeatgLvlSts_DrvrSeatHeatgLvlSts_read)
  DrvrSeatHeatgLvlSts_DrvrSeatHeatgLvlSts_IsUpdated/1 -> PrkgClimaActvMgr_Runnable_sys/134(DrvrSeatHeatgLvlSts_DrvrSeatHeatgLvlSts_IsUpdated_read)
  DrvrSeatVentnLvlSts_DrvrSeatVentnLvlSts/1 -> PrkgClimaActvMgr_Runnable_sys/135(DrvrSeatVentnLvlSts_DrvrSeatVentnLvlSts_read)
  DrvrSeatVentnLvlSts_DrvrSeatVentnLvlSts_IsUpdated/1 -> PrkgClimaActvMgr_Runnable_sys/136(DrvrSeatVentnLvlSts_DrvrSeatVentnLvlSts_IsUpdated_read)
  DrvrSeatVentnLvlSts2Ngcc_DrvrSeatVentnLvlSts2Ngcc/1 -> PrkgClimaActvMgr_Runnable_sys/137(DrvrSeatVentnLvlSts2Ngcc_DrvrSeatVentnLvlSts2Ngcc_read)
  EngSt1WdSts_Ngcc_EngSt1WdSts/1 -> PrkgClimaActvMgr_Runnable_sys/138(EngSt1WdSts_Ngcc_EngSt1WdSts_read)
  ErsCmdByKeyFromExsrc_ErsCmdByKeyFromExsrc/1 -> PrkgClimaActvMgr_Runnable_sys/139(ErsCmdByKeyFromExsrc_ErsCmdByKeyFromExsrc_read)
  GearLvrIndcn_GearLvrIndcn/1 -> PrkgClimaActvMgr_Runnable_sys/140(GearLvrIndcn_GearLvrIndcn_read)
  GearLvrIndcn_GearLvrIndcn_IsUpdated/1 -> PrkgClimaActvMgr_Runnable_sys/141(GearLvrIndcn_GearLvrIndcn_IsUpdated_read)
  HighTempCleanHmiReqNgcc_HighTempCleanHmiReqNgcc/1 -> PrkgClimaActvMgr_Runnable_sys/142(HighTempCleanHmiReqNgcc_HighTempCleanHmiReqNgcc_read)
  HMIAirCdnrAutDrySetNgcc_HMIAirCdnrAutDrySetNgcc/1 -> PrkgClimaActvMgr_Runnable_sys/143(HMIAirCdnrAutDrySetNgcc_HMIAirCdnrAutDrySetNgcc_read)
  HMIAirCdnrAutDrySetNgcc_HMIAirCdnrAutDrySetNgcc_IsUpdated/1 -> PrkgClimaActvMgr_Runnable_sys/144(HMIAirCdnrAutDrySetNgcc_HMIAirCdnrAutDrySetNgcc_IsUpdated_read)
  HmiCmptmtTSp_HmiCmptmtTSp/1 -> PrkgClimaActvMgr_Runnable_sys/145(HmiCmptmtTSp_HmiCmptmtTSp_read)
  HMILBRACStrtSetNgcc_HMILBRACStrtSetNgcc/1 -> PrkgClimaActvMgr_Runnable_sys/146(HMILBRACStrtSetNgcc_HMILBRACStrtSetNgcc_read)
  HmiParkVentSetNgcc_HmiParkVentSetNgcc/1 -> PrkgClimaActvMgr_Runnable_sys/147(HmiParkVentSetNgcc_HmiParkVentSetNgcc_read)
  HmiParkVentTmrSetNgcc_HmiParkVentTmrSetNgcc/1 -> PrkgClimaActvMgr_Runnable_sys/148(HmiParkVentTmrSetNgcc_HmiParkVentTmrSetNgcc_read)
  HvBattMntnActv_HvBattMntnActv/1 -> PrkgClimaActvMgr_Runnable_sys/149(HvBattMntnActv_HvBattMntnActv_read)
  HvCooltHeatrStsSig_HvCooltHeatrStsSig/1 -> PrkgClimaActvMgr_Runnable_sys/150(HvCooltHeatrStsSig_HvCooltHeatrStsSig_read)
  HvCooltHeatrStsSig_HvCooltHeatrStsSig_IsUpdated/1 -> PrkgClimaActvMgr_Runnable_sys/151(HvCooltHeatrStsSig_HvCooltHeatrStsSig_IsUpdated_read)
  IntPm25VluFrmClima_IntPm25VluFrmClima/1 -> PrkgClimaActvMgr_Runnable_sys/152(IntPm25VluFrmClima_IntPm25VluFrmClima_read)